simulink model "pasp_4i16c1ks"  (Simulink 7.3)
settings: Solver=ode45  StartTime=0.0  StopTime=10  RelTol=1e-3
undefined symbols (not defined in the file or in its folder): dist_gbe, ip_ctr, makereorderarray, pasp_lib, pfb_coeff_gen_calc, ROACH
libraries: xbsIndex_r4 x2298, casper_library x259, xps_library x35, gavrt_library x2
(part 5 of 27)

== system pasp_4i16c1ks/pasp/scope_output6 ==
  rst : Inport  OutDataType=sfix(16)  OutScaling=2^0
  en : Inport  Port=2  OutDataType=sfix(16)  OutScaling=2^0
  data_in : Inport  Port=3  OutDataType=sfix(16)  OutScaling=2^0
  bram : xps_library/Shared BRAM  Tag=xps:bram  SystemSampleTime=-1  arith_type="Signed  (2's comp)"  addr_width=11  data_width=32  data_bin_pt=17  init_vals=[1:2^11]  sample_rate=1
  freeze_cntr : casper_library/Misc/freeze_cntr  SystemSampleTime=-1  CounterBits=11
  wires:
  freeze_cntr/1 -> bram/1
  freeze_cntr/2 -> bram/3
  en/1 -> freeze_cntr/1
  data_in/1 -> bram/2
  rst/1 -> freeze_cntr/2

== system pasp_4i16c1ks/pasp/scope_output7 ==
  rst : Inport  OutDataType=sfix(16)  OutScaling=2^0
  en : Inport  Port=2  OutDataType=sfix(16)  OutScaling=2^0
  data_in : Inport  Port=3  OutDataType=sfix(16)  OutScaling=2^0
  bram : xps_library/Shared BRAM  Tag=xps:bram  SystemSampleTime=-1  arith_type="Signed  (2's comp)"  addr_width=11  data_width=32  data_bin_pt=17  init_vals=[1:2^11]  sample_rate=1
  freeze_cntr : casper_library/Misc/freeze_cntr  SystemSampleTime=-1  CounterBits=11
  wires:
  rst/1 -> freeze_cntr/2
  data_in/1 -> bram/2
  en/1 -> freeze_cntr/1
  freeze_cntr/2 -> bram/3
  freeze_cntr/1 -> bram/1

== system pasp_4i16c1ks/pasp/scope_output8 ==
  rst : Inport  OutDataType=sfix(16)  OutScaling=2^0
  en : Inport  Port=2  OutDataType=sfix(16)  OutScaling=2^0
  data_in : Inport  Port=3  OutDataType=sfix(16)  OutScaling=2^0
  bram : xps_library/Shared BRAM  Tag=xps:bram  SystemSampleTime=-1  arith_type="Signed  (2's comp)"  addr_width=11  data_width=32  data_bin_pt=17  init_vals=[1:2^11]  sample_rate=1
  freeze_cntr : casper_library/Misc/freeze_cntr  SystemSampleTime=-1  CounterBits=11
  wires:
  freeze_cntr/1 -> bram/1
  freeze_cntr/2 -> bram/3
  en/1 -> freeze_cntr/1
  data_in/1 -> bram/2
  rst/1 -> freeze_cntr/2

== system pasp_4i16c1ks/pasp/sync_generator ==
  period : Inport  OutDataType=sfix(16)  OutScaling=2^0
  reset : Inport  Port=2  OutDataType=sfix(16)  OutScaling=2^0
  Convert : xbsIndex_r4/Convert  arith_type=Boolean  n_bits=8  bin_pt=6  quantization=Truncate  overflow=Wrap  latency=1  dbl_ovrd=off  pipeline=off  block_type=convert
      settings: xl_area=[0, 0, 0, 0, 0, 0, 0]  block_version=10.1.3
  Counter : xbsIndex_r4/Counter  cnt_type=Free Running  cnt_to=Inf  operation=Up  start_count=1  cnt_by_val=1  arith_type=Unsigned  n_bits=32  bin_pt=0  load_pin=off  rst=on  en=off  explicit_period=on  period=1  dbl_ovrd=off  use_behavioral_HDL=off  implementation=Fabric  use_rpm=off  block_type=counter
  Logical : xbsIndex_r4/Logical  logical_function=OR  inputs=2  en=off  latency=0  precision=Full  arith_type=Unsigned  n_bits=8  bin_pt=2  align_bp=on  dbl_ovrd=off  block_type=logical
      settings: xl_area=[0, 0, 0, 0, 0, 0, 0]
  Relational : xbsIndex_r4/Relational  mode=a>=b  en=off  latency=1  dbl_ovrd=off  block_type=relational
      settings: xl_area=[0, 0, 0, 0, 0, 0, 0]  block_version=10.1.3
  cns : xbsIndex_r4/Constant  arith_type=Unsigned  const=4  n_bits=3  bin_pt=0  explicit_period=off  period=1  equ=P=C  opselect=C  inp2=PCIN>>17  opr=+  inp1=P  carry=CIN  dbl_ovrd=off  block_type=constant
  period_sub : xbsIndex_r4/AddSub  mode=Subtraction  use_carryin=off  use_carryout=off  en=off  latency=0  precision=User Defined  arith_type=Unsigned  n_bits=32  bin_pt=0  quantization=Truncate  overflow=Wrap  dbl_ovrd=off  use_behavioral_HDL=on  hw_selection=Fabric  pipelined=off  use_rpm=on  block_type=addsub
      settings: xl_area=[0, 0, 0, 0, 0, 0, 0]  block_version=10.1.3
  posedge : casper_library/Misc/posedge  Tag=Rising Edge Detector  SystemSampleTime=-1
  sync : Outport  OutDataType=sfix(16)  OutScaling=2^0
  wires:
  Counter/1 -> Relational/1
  Relational/1 -> Convert/1
  Convert/1 -> Logical/2
  Logical/1 -> posedge/1, Counter/1
  reset/1 -> Logical/1
  period/1 -> period_sub/1
  period_sub/1 -> Relational/2
  cns/1 -> period_sub/2
  posedge/1 -> sync/1

== system pasp_4i16c1ks/pasp/dist_gbe/ip_ctr ==
  Count : Inport
  Reset : Inport  Port=2  OutDataType=sfix(16)  OutScaling=2^0
  Compareto0 : xbsIndex_r4/Relational  mode=a=b  en=off  latency=1  dbl_ovrd=off  block_type=relational
  Counter : xbsIndex_r4/Counter  cnt_type=Free Running  cnt_to=Inf  operation=Up  start_count=0  cnt_by_val=1  arith_type=Unsigned  n_bits=2  bin_pt=0  load_pin=off  rst=on  en=on  explicit_period=on  period=1  dbl_ovrd=off  use_behavioral_HDL=off  implementation=Fabric  block_type=counter
      settings: block_version=11.4
  IPMux : xbsIndex_r4/Mux  inputs=4  en=off  latency=3  precision=Full  arith_type=Unsigned  n_bits=16  bin_pt=14  quantization=Truncate  overflow=Wrap  dbl_ovrd=off  block_type=mux
      settings: block_version=11.4
  IP_Constant0 : Constant  Value=4001  OutDataType=fixdt(1, 16)  OutScaling=2^0
  IP_Constant1 : Constant  Value=4002  OutDataType=sfix(16)  OutScaling=2^0
  IP_Constant2 : Constant  Value=4003  OutDataType=sfix(16)  OutScaling=2^0
  IP_Constant3 : Constant  Value=4004  OutDataType=sfix(16)  OutScaling=2^0
  PortMux : xbsIndex_r4/Mux  inputs=4  en=off  latency=3  precision=Full  arith_type=Unsigned  n_bits=16  bin_pt=14  quantization=Truncate  overflow=Wrap  dbl_ovrd=off  block_type=mux
      settings: block_version=11.4
  PortSlice : xbsIndex_r4/Slice  nbits=16  boolean_output=off  mode=Lower Bit Location + Width  bit1=0  base1=MSB of Input  bit0=0  base0=LSB of Input  dbl_ovrd=off  block_type=slice
  Port_Constant0 : Constant  Value=4000  OutDataType=fixdt(1, 16)  OutScaling=2^0
  Port_Constant1 : Constant  Value=4000  OutDataType=sfix(16)  OutScaling=2^0
  Port_Constant2 : Constant  Value=4000  OutDataType=sfix(16)  OutScaling=2^0
  Port_Constant3 : Constant  Value=4000  OutDataType=sfix(16)  OutScaling=2^0
  const_0 : xbsIndex_r4/Constant  arith_type=Unsigned  const=0  n_bits=32  bin_pt=0  explicit_period=off  period=1  equ=P=C  opselect=C  inp2=PCIN>>17  opr=+  inp1=P  carry=CIN  dbl_ovrd=off  block_type=constant
      settings: block_version=11.4
  reg_ip0 : xps_library/software register  Tag=xps:sw_reg  SystemSampleTime=-1  io_dir=From Processor  arith_type=Unsigned  bitwidth=32  bin_pt=0  sample_period=1  gw_name=pasp_4i16c1ks_pasp_dist_gbe_ip_ctr_reg_ip0_user_data_out
  reg_ip1 : xps_library/software register  Tag=xps:sw_reg  SystemSampleTime=-1  io_dir=From Processor  arith_type=Unsigned  bitwidth=32  bin_pt=0  sample_period=1  gw_name=pasp_4i16c1ks_pasp_dist_gbe_ip_ctr_reg_ip1_user_data_out
      link override pasp_16i16c128s_pasp_dist_gbe_ip_ctr_reg_ip1_user_data_out: arith_type=Unsigned  n_bits=32  bin_pt=0  period=1
  reg_ip2 : xps_library/software register  Tag=xps:sw_reg  SystemSampleTime=-1  io_dir=From Processor  arith_type=Unsigned  bitwidth=32  bin_pt=0  sample_period=1  gw_name=pasp_4i16c1ks_pasp_dist_gbe_ip_ctr_reg_ip2_user_data_out
      link override pasp_16i16c128s_pasp_dist_gbe_ip_ctr_reg_ip2_user_data_out: arith_type=Unsigned  n_bits=32  bin_pt=0  period=1
  reg_ip3 : xps_library/software register  Tag=xps:sw_reg  SystemSampleTime=-1  io_dir=From Processor  arith_type=Unsigned  bitwidth=32  bin_pt=0  sample_period=1  gw_name=pasp_4i16c1ks_pasp_dist_gbe_ip_ctr_reg_ip3_user_data_out
      link override pasp_16i16c128s_pasp_dist_gbe_ip_ctr_reg_ip3_user_data_out: arith_type=Unsigned  n_bits=32  bin_pt=0  period=1
  reg_port0 : xps_library/software register  Tag=xps:sw_reg  SystemSampleTime=-1  io_dir=From Processor  arith_type=Unsigned  bitwidth=32  bin_pt=0  sample_period=1  gw_name=pasp_4i16c1ks_pasp_dist_gbe_ip_ctr_reg_port0_user_data_out
  reg_port1 : xps_library/software register  Tag=xps:sw_reg  SystemSampleTime=-1  io_dir=From Processor  arith_type=Unsigned  bitwidth=32  bin_pt=0  sample_period=1  gw_name=pasp_4i16c1ks_pasp_dist_gbe_ip_ctr_reg_port1_user_data_out
      link override pasp_16i16c128s_pasp_dist_gbe_ip_ctr_reg_port1_user_data_out: arith_type=Unsigned  n_bits=32  bin_pt=0  period=1
  reg_port2 : xps_library/software register  Tag=xps:sw_reg  SystemSampleTime=-1  io_dir=From Processor  arith_type=Unsigned  bitwidth=32  bin_pt=0  sample_period=1  gw_name=pasp_4i16c1ks_pasp_dist_gbe_ip_ctr_reg_port2_user_data_out
      link override pasp_16i16c128s_pasp_dist_gbe_ip_ctr_reg_port2_user_data_out: arith_type=Unsigned  n_bits=32  bin_pt=0  period=1
  reg_port3 : xps_library/software register  Tag=xps:sw_reg  SystemSampleTime=-1  io_dir=From Processor  arith_type=Unsigned  bitwidth=32  bin_pt=0  sample_period=1  gw_name=pasp_4i16c1ks_pasp_dist_gbe_ip_ctr_reg_port3_user_data_out
      link override pasp_16i16c128s_pasp_dist_gbe_ip_ctr_reg_port3_user_data_out: arith_type=Unsigned  n_bits=32  bin_pt=0  period=1
  IP : Outport
  Port : Outport  Port=2
  Discard_pkt : Outport  Port=3
  wires:
  Reset/1 -> Counter/1
  Count/1 -> Counter/2
  Counter/1 -> IPMux/1, PortMux/1
  IPMux/1 -> IP/1, Compareto0/2
  PortMux/1 -> PortSlice/1
  PortSlice/1 -> Port/1
  const_0/1 -> Compareto0/1
  Compareto0/1 -> Discard_pkt/1
  IP_Constant0/1 -> reg_ip0/1
  reg_ip0/1 -> IPMux/2
  Port_Constant0/1 -> reg_port0/1
  reg_port0/1 -> PortMux/2
  IP_Constant1/1 -> reg_ip1/1
  reg_ip1/1 -> IPMux/3
  Port_Constant1/1 -> reg_port1/1
  reg_port1/1 -> PortMux/3
  IP_Constant2/1 -> reg_ip2/1
  reg_ip2/1 -> IPMux/4
  Port_Constant2/1 -> reg_port2/1
  reg_port2/1 -> PortMux/4
  IP_Constant3/1 -> reg_ip3/1
  reg_ip3/1 -> IPMux/5
  Port_Constant3/1 -> reg_port3/1
  reg_port3/1 -> PortMux/5

== system pasp_4i16c1ks/pasp/dist_gbe/reorder ==
  sync : Inport  OutDataType=sfix(16)  OutScaling=2^0
  en : Inport  Port=2  OutDataType=sfix(16)  OutScaling=2^0
  din0 : Inport  Port=3  OutDataType=sfix(16)  OutScaling=2^0
  Counter : xbsIndex_r4/Counter  cnt_type=Count Limited  cnt_to=8191  operation=Up  start_count=0  cnt_by_val=1  arith_type=Unsigned  n_bits=13  bin_pt=0  load_pin=off  rst=on  en=on  explicit_period=on  period=1  dbl_ovrd=off  use_behavioral_HDL=off  implementation=Fabric  block_type=counter
      settings: block_version=11.4
  Slice1 : xbsIndex_r4/Slice  nbits=1  boolean_output=off  mode=Upper Bit Location + Width  bit1=0  base1=MSB of Input  bit0=0  base0=LSB of Input  dbl_ovrd=off  block_type=slice
  Slice2 : xbsIndex_r4/Slice  nbits=12  boolean_output=off  mode=Lower Bit Location + Width  bit1=0  base1=MSB of Input  bit0=0  base0=LSB of Input  dbl_ovrd=off  block_type=slice
  dbl_buffer0 : casper_library/Reorder/dbl_buffer  SystemSampleTime=-1  depth=4096  latency=2
  delay_d0 : xbsIndex_r4/Delay  en=off  latency=3  dbl_ovrd=off  reg_retiming=off  block_type=delay
      settings: block_version=11.4
  delay_din0 : xbsIndex_r4/Delay  en=off  latency=3  dbl_ovrd=off  reg_retiming=off  block_type=delay
      settings: xl_area=[0, 0, 0, 0, 0, 0, 0]  block_version=9.1.01
  delay_sel : xbsIndex_r4/Delay  en=off  latency=3  dbl_ovrd=off  reg_retiming=off  block_type=delay
      settings: block_version=11.4
  delay_valid : xbsIndex_r4/Delay  en=off  latency=4  dbl_ovrd=off  reg_retiming=off  block_type=delay
      settings: xl_area=[0, 0, 0, 0, 0, 0, 0]  block_version=9.1.01
  delay_we : xbsIndex_r4/Delay  en=off  latency=3  dbl_ovrd=off  reg_retiming=off  block_type=delay
      settings: xl_area=[0, 0, 0, 0, 0, 0, 0]  block_version=9.1.01
  map1 : xbsIndex_r4/ROM  depth=4096  initVector=[0 1 8 9 16 17 24 25 32 33 40 41 48 49 56 57 64 65 72 73 80 81 88 89 96 97 104 105 112 113 120 121 128 129 136 137 144 145 152 153 160 161 168 169 176 177 184 185 192 193 200 201 208 209 216 217 224 225 232 233 240 241 248 249 256 257 264 265 272 273 280 281 288 289 296 297 304 305 312 313 320 321 328 329 336 337 344 345 352 353 360 361 368 369 376 377 384 385 392 393 400 401 408 409 416 417 424 425 432 433 440 441 448 449 456 457 464 465 472 473 480 481 488 489 496 497 504 505 512 513 520 521 528 529 536 537 544 545 552 553 560 561 568 569 576 577 584 585 592 593 600 601 608 609 616 617 624 625 632 633 640 641 648 649 656 657 664 665 672 673 680 681 688 689 696 697 704 705 712 713 720 721 728 729 736 737 744 745 752 753 760 761 768 769 776 777 784 785 792 793 800 801 808 809 816 817 824 825 832 833 840 841 848 849 856 857 864 865 872 873 880 881 888 889 896 897 904 905 912 913 920 921 928 929 936 937 944 945 952 953 960 961 968 969 976 977 984 985 992 993 1000 1001 1008 1009 1016 1017 1024 1025 1032 1033 1040 1041 1048 1049 1056 1057 1064 1065 1072 1073 1080 1081 1088 1089 1096 1097 1104 1105 1112 1113 1120 1121 1128 1129 1136 1137 1144 1145 1152 1153 1160 1161 1168 1169 1176 1177 1184 1185 1192 1193 1200 1201 1208 1209 1216 1217 1224 1225 1232 1233 1240 1241 1248 1249 1256 1257 1264 1265 1272 1273 1280 1281 1288 1289 1296 1297 1304 1305 1312 1313 1320 1321 1328 1329 1336 1337 1344 1345 1352 1353 1360 1361 1368 1369 1376 1377 1384 1385 1392 1393 1400 1401 1408 1409 1416 1417 1424 1425 1432 1433 1440 1441 1448 1449 1456 1457 1464 1465 1472 1473 1480 1481 1488 1489 1496 1497 1504 1505 1512 1513 1520 1521 1528 1529 1536 1537 1544 1545 1552 1553 1560 1561 1568 1569 1576 1577 1584 1585 1592 1593 1600 1601 1608 1609 1616 1617 1624 1625 1632 1633 1640 1641 1648 1649 1656 1657 1664 1665 1672 1673 1680 1681 1688 1689 1696 1697 1704 1705 1712 1713 1720 1721 1728 1729 1736 1737 1744 1745 1752 1753 1760 1761 1768 1769 1776 1777 1784 1785 1792 1793 1800 1801 1808 1809 1816 1817 1824 1825 1832 1833 1840 1841 1848 1849 1856 1857 1864 1865 1872 1873 1880 1881 1888 1889 1896 1897 1904 1905 1912 1913 1920 1921 1928 1929 1936 1937 1944 1945 1952 1953 1960 1961 1968 1969 1976 1977 1984 1985 1992 1993 2000 2001 2008 2009 2016 2017 2024 2025 2032 2033 2040 2041 2048 2049 2056 2057 2064 2065 2072 2073 2080 2081 2088 2089 2096 2097 2104 2105 2112 2113 2120 2121 2128 2129 2136 2137 2144 2145 2152 2153 2160 2161 2168 2169 2176 2177 2184 2185 2192 2193 2200 2201 2208 2209 2216 2217 2224 2225 2232 2233 2240 2241 2248 2249 2256 2257 2264 2265 2272 2273 2280 2281 2288 2289 2296 2297 2304 2305 2312 2313 2320 2321 2328 2329 2336 2337 2344 2345 2352 2353 2360 2361 2368 2369 2376 2377 2384 2385 2392 2393 2400 2401 2408 2409 2416 2417 2424 2425 2432 2433 2440 2441 2448 2449 2456 2457 2464 2465 2472 2473 2480 2481 2488 2489 2496 2497 2504 2505 2512 2513 2520 2521 2528 2529 2536 2537 2544 2545 2552 2553 2560 2561 2568 2569 2576 2577 2584 2585 2592 2593 2600 2601 2608 2609 2616 2617 2624 2625 2632 2633 2640 2641 2648 2649 2656 2657 2664 2665 2672 2673 2680 2681 2688 2689 2696 2697 2704 2705 2712 2713 2720 2721 2728 2729 2736 2737 2744 2745 2752 2753 2760 2761 2768 2769 2776 2777 2784 2785 2792 2793 2800 2801 2808 2809 2816 2817 2824 2825 2832 2833 2840 2841 2848 2849 2856 2857 2864 2865 2872 2873 2880 2881 2888 2889 2896 2897 2904 2905 2912 2913 2920 2921 2928 2929 2936 2937 2944 2945 2952 2953 2960 2961 2968 2969 2976 2977 2984 2985 2992 2993 3000 3001 3008 3009 3016 3017 3024 3025 3032 3033 3040 3041 3048 3049 3056 3057 3064 3065 3072 3073 3080 3081 3088 3089 3096 3097 3104 3105 3112 3113 3120 3121 3128 3129 3136 3137 3144 3145 3152 3153 3160 3161 3168 3169 3176 3177 3184 3185 3192 3193 3200 3201 3208 3209 3216 3217 3224 3225 3232 3233 3240 3241 3248 3249 3256 3257 3264 3265 3272 3273 3280 3281 3288 3289 3296 3297 3304 3305 3312 3313 3320 3321 3328 3329 3336 3337 3344 3345 3352 3353 3360 3361 3368 3369 3376 3377 3384 3385 3392 3393 3400 3401 3408 3409 3416 ...(+15375 chars)]  distributed_mem=Distributed memory  rst=off  init_reg=0  en=off  latency=3  arith_type=Unsigned  n_bits=12  bin_pt=0  dbl_ovrd=off  optimize=Area  use_rpm=on  block_type=sprom
      settings: block_version=11.4
  post_sync_delay : xbsIndex_r4/Delay  en=off  latency=4  dbl_ovrd=off  reg_retiming=off  block_type=delay
      settings: xl_area=[0, 0, 0, 0, 0, 0, 0]  block_version=9.1.01
  pre_sync_delay : xbsIndex_r4/Delay  en=off  latency=3  dbl_ovrd=off  reg_retiming=off  block_type=delay
      settings: xl_area=[0, 0, 0, 0, 0, 0, 0]  block_version=9.1.01
  sync_delay_en : casper_library/Delays/sync_delay_en  SystemSampleTime=-1  DelayLen=4096
  sync_out : Outport  OutDataType=sfix(16)  OutScaling=2^0
  valid : Outport  Port=2  OutDataType=sfix(16)  OutScaling=2^0
  dout0 : Outport  Port=3  OutDataType=sfix(16)  OutScaling=2^0
  wires:
  sync/1 -> Counter/1, pre_sync_delay/1
  en/1 -> Counter/2, sync_delay_en/2, delay_we/1
  Counter/1 -> Slice1/1, Slice2/1
  Slice1/1 -> delay_sel/1
  Slice2/1 -> delay_d0/1, map1/1
  pre_sync_delay/1 -> sync_delay_en/1
  sync_delay_en/1 -> post_sync_delay/1
  post_sync_delay/1 -> sync_out/1
  delay_we/1 -> delay_valid/1, dbl_buffer0/5
  delay_valid/1 -> valid/1
  delay_d0/1 -> dbl_buffer0/2
  map1/1 -> dbl_buffer0/3
  delay_sel/1 -> dbl_buffer0/1
  din0/1 -> delay_din0/1
  delay_din0/1 -> dbl_buffer0/4
  dbl_buffer0/1 -> dout0/1

== system pasp_4i16c1ks/pasp/fft_wideband_real/fft_biplex_real_4x0 ==
  pol1 : Inport  OutDataType=sfix(16)  OutScaling=2^0
  pol2 : Inport  Port=2  OutDataType=sfix(16)  OutScaling=2^0
  pol3 : Inport  Port=3  OutDataType=sfix(16)  OutScaling=2^0
  pol4 : Inport  Port=4  OutDataType=sfix(16)  OutScaling=2^0
  sync : Inport  Port=5  OutDataType=sfix(16)  OutScaling=2^0
  shift : Inport  Port=6  OutDataType=sfix(16)  OutScaling=2^0
  bi_real_unscr_4x : Subsystem  mask{FFTSize=3, bram_latency=2}
      mask prompts: FFTSize "Size of FFT (2^?):"; bram_latency "BRAM Latency"
      mask init:
      | blk = gcb;
      | if same_state(blk, 'FFTSize', FFTSize, 'bram_latency', bram_latency), return, end
      | set_param([blk,'/reorder'],'map', mat2str(bit_reverse(0:2^(FFTSize-1)-1, FFTSize-1)));
      | set_param([blk,'/reorder'],'bram_latency', num2str(bram_latency));
      | set_param([blk,'/reorder1'],'map', mat2str(bit_reverse(2^(FFTSize-1)-1:-1:0, FFTSize-1)));
      | set_param([blk,'/reorder1'],'bram_latency', num2str(bram_latency));
      | set_param([blk,'/reorder2'],'map', mat2str(2^(FFTSize-1)-1:-1:0));
      | set_param([blk,'/reorder2'],'bram_latency', num2str(bram_latency));
      | save_state(blk, 'FFTSize', FFTSize, 'bram_latency', bram_latency);
  biplex_core : Subsystem  AncestorBlock=casper_library/FFTs/biplex_core  mask(biplex_core){FFTSize=3, input_bit_width=18, coeff_bit_width=18, quantization=Truncate, overflow=Wrap, add_latency=2, mult_latency=3, bram_latency=2, arch=Virtex2Pro, opt_target=Multipliers, coeffs_bit_limit=8, delays_bit_limit=9, specify_mult=off, mult_spec=[2 2 2], dsp48_adders=off}
      mask prompts: FFTSize "Size of FFT: (2^? pnts)"; input_bit_width "Input/Output Bit Width"; coeff_bit_width "Coefficient Bit Width"; quantization "Quantization Behavior"; overflow "Overflow Behavior"; add_latency "Add Latency"; mult_latency "Mult Latency"; bram_latency "BRAM Latency"; arch "Architecture"; opt_target "Optimisation Target"; coeffs_bit_limit "Number bits above which to store stage's coefficients in BRAM (2^? bits)"; delays_bit_limit "Number bits above which to implent stage's delays in BRAM (2^? bits)"; specify_mult "Specify multiplier use (behavioural HDL if not specified)"; mult_spec "Multiplier specification (0=core, 1=embedded, 2=behavioural) (left=1st stage)"; dsp48_adders "Use DSP48s for adders"
      mask init:
      | biplex_core_init(gcb,...
      |     'FFTSize', FFTSize,...
      |     'input_bit_width', input_bit_width,...
      |     'coeff_bit_width', coeff_bit_width,...
      |     'quantization', quantization,...
      |     'overflow', overflow,...
      |     'add_latency', add_latency,...
      |     'mult_latency', mult_latency,...
      |     'bram_latency', bram_latency,...
      |     'arch', arch, ...
      |     'opt_target', opt_target, ...
      |     'coeffs_bit_limit', coeffs_bit_limit, ...
      |     'delays_bit_limit', delays_bit_limit, ...
      |     'specify_mult', specify_mult, ...
      |     'mult_spec', mult_spec, ...
      |     'dsp48_adders', dsp48_adders );
      settings: AttributesFormatString=|
      | FFTSize=3,
      |  opt_target=Multipliers,
      |  arch=Virtex2Pro
      mask StyleString: edit,edit,edit,popup(Truncate|Round  (unbiased: +/- Inf)|Round  (unbiased: Even Values)),popup(Wrap|Saturate|Error),edit,edit,edit,popup(Virtex2Pro|Virtex5),popup(Logic|Multipliers),edit,edit,checkbox,edit,checkbox
      mask CallbackString:
      | % fft_size = eval(get_param(gcb, 'FFTSize'));
      | % old_vec = eval(get_param(gcb, 'mult_spec'));
      | 
      | % vec = 2.*ones(1,fft_size);
      | % old_size = length(old_vec);
      | 
      | % if(old_size <= fft_size),
      | %    size = old_size;
      | % else,
      | %     size = fft_size;
      | % end
      | 
      | % for n = 1:size, vec(n) = old_vec(n); end
      | % set_param(gcb, 'mult_spec', mat2str(vec));||||||||||||specify_mult = get_param(gcb,'specify_mult');
      | visibs = get_param(gcb,'MaskVisibilities');
      | if( strcmp(specify_mult,'off')), visibs{14} = 'off';
      | else, visibs{14} = 'on';
      | end
      | set_param(gcb, 'MaskVisibilities', visibs);
      | enables = get_param(gcb,'MaskVisibilities');
      | enables{14} = 'on';
      | set_param(gcb, 'MaskEnables', enables);
      | |% vec = eval( get_param(gcb, 'mult_spec'));
      | % vec_size = length(vec);
      | % fft_size = eval( get_param(gcb, 'FFTSize'));
      | % new_vec = 2.*ones(1, fft_size);
      | 
      | % if(vec_size <= fft_size), size = vec_size;
      | % else size = fft_size;
      | % end
      | 
      | % for n=1:size
      | %     new_vec(n) = vec(n);
      | % end
      | % set_param(gcb, 'mult_spec', mat2str(new_vec));|
  ri_to_c : Subsystem
  ri_to_c1 : Subsystem
  pol1_out : Outport  OutDataType=sfix(16)  OutScaling=2^0
  pol2_out : Outport  Port=2  OutDataType=sfix(16)  OutScaling=2^0
  pol3_out : Outport  Port=3  OutDataType=sfix(16)  OutScaling=2^0
  pol4_out : Outport  Port=4  OutDataType=sfix(16)  OutScaling=2^0
  of : Outport  Port=5  OutDataType=sfix(16)  OutScaling=2^0
  sync_out : Outport  Port=6  OutDataType=sfix(16)  OutScaling=2^0
  wires:
  bi_real_unscr_4x/5(sync_out) -> sync_out/1
  bi_real_unscr_4x/4(pol4_out) -> pol4_out/1
  bi_real_unscr_4x/3(pol3_out) -> pol3_out/1
  bi_real_unscr_4x/2(pol2_out) -> pol2_out/1
  bi_real_unscr_4x/1(pol1_out) -> pol1_out/1
  shift/1 -> biplex_core/4(shift)
  sync/1 -> biplex_core/3(sync)
  pol4/1 -> ri_to_c1/2(im)
  ri_to_c1/1(c) -> biplex_core/2(pol2)
  pol3/1 -> ri_to_c1/1(re)
  pol2/1 -> ri_to_c/2(im)
  ri_to_c/1(c) -> biplex_core/1(pol1)
  pol1/1 -> ri_to_c/1(re)
  biplex_core/3(of) -> of/1
  biplex_core/1(out1) -> bi_real_unscr_4x/1(even)
  biplex_core/2(out2) -> bi_real_unscr_4x/2(odd)
  biplex_core/4(sync_out) -> bi_real_unscr_4x/3(sync)
